simulink model "wlan_phy_rx_pmd"  (Simulink 7.8)
settings: Solver=VariableStepDiscrete  SolverMode=SingleTasking  StartTime=0.0  StopTime=rx_sim_time  RelTol=1e-3
PostLoadFcn:
  | wlan_phy_rx_init
InitFcn:
  | wlan_phy_rx_init
StartFcn:
  | wlan_phy_rx_init
values from neighbouring files (symbols this model uses):
  barker_seq20 = 0.95 * circshift(barker_seq20, [0 4]) ./ max(abs(barker_seq20))    [wlan_phy_rx_init.m]
  bit_scrambler_lfsr_bytes = sum(bit_scrambler_lfsr_bytes .* repmat(2.^[0:7], 127, 1),2)    [wlan_phy_rx_init.m]
  deinterleave_ROM = [deinterleave_ROM interleave_11n_64QAM zeros(1, 512-length(interleave_11n_64QAM))]    [wlan_phy_rx_init.m]
  longCorr_coef_i = [3*real(longCorr_coef)]    [wlan_phy_rx_init.m]
  longCorr_coef_q = [3*imag(longCorr_coef)]    [wlan_phy_rx_init.m]
  MAX_CP_LEN = 32    [wlan_phy_rx_init.m]
  MAX_NCBPS = NCBPS_11n_64QAM    (= 312)    [wlan_phy_rx_init.m]
  MAX_NUM_BYTES = 2^16-1    (= 65535)    [wlan_phy_rx_init.m]
  MAX_NUM_SC = 64    [wlan_phy_rx_init.m]
  MAX_NUM_SYMS = 600    [wlan_phy_rx_init.m]
  NCBPS_11a_BPSK = 48    [wlan_phy_rx_init.m]
  NDBPS_11a_BPSK12 = 24    [wlan_phy_rx_init.m]
  PHY_SIGNAL_MIN_LEN = 14    [wlan_phy_rx_init.m]
  preFFT_sampBuff_numSamps = 4*MAX_NUM_SC    (= 256)    [wlan_phy_rx_init.m]
  rx_sim_sig_samp_time = 8    [wlan_phy_rx_init.m]
  scr_ind_rev = zeros(1,128)    [wlan_phy_rx_init.m]
undefined symbols (not defined in the file or in its folder): gcbh, mcs_rom_11ag, mcs_rom_11n, xlBlockLoadCallback, xlGetNormalizedPeriod, xlipgetpartsetting
libraries: xbsIndex_r4 x3862, simulink x1, xbsEDKLib_r4 x1
(part 5 of 23)

== system wlan_phy_rx_pmd/DSSS Rx/SIGNAL Dec ==
  note: LENGTH*8 + 6 - 1:
      | Length in usec = Number of bits
      | *8: Convert to bytes
      | +6: Add SIGNAL, SERVICE and CRC bytes
      | -1: Downstream logic requires zero-indexed length
      | to compare vs. byte index
  Byte : Inport
  Byte Valid : Inport  Port=2
  Byte Ind : Inport  Port=3
  CRC Calc : Inport  Port=4
  AddSub : xbsIndex_r4/AddSub  mode=Addition  use_carryin=off  use_carryout=off  en=off  latency=0  precision=User Defined  arith_type=Unsigned  n_bits=ceil(log2(MAX_NUM_BYTES))  bin_pt=0  quantization=Truncate  overflow=Wrap  dbl_ovrd=off  use_behavioral_HDL=off  hw_selection=Fabric  pipelined=off  block_type=addsub
  Concat : xbsIndex_r4/Concat  num_inputs=2  dbl_ovrd=off  block_type=concat
  Concat1 : xbsIndex_r4/Concat  num_inputs=2  dbl_ovrd=off  block_type=concat
  Constant1 : xbsIndex_r4/Constant  const=2  gui_display_data_type=Fixed-point  arith_type=Unsigned  n_bits=2  bin_pt=0  preci_type=Single  exp_width=8  frac_width=24  explicit_period=off  period=1  equ=P=C  opselect=C  inp2=PCIN>>17  opr=+  inp1=P  carry=CIN  dbl_ovrd=off  block_type=constant
  Constant2 : xbsIndex_r4/Constant  const=0  gui_display_data_type=Fixed-point  arith_type=Unsigned  n_bits=2  bin_pt=0  preci_type=Single  exp_width=8  frac_width=24  explicit_period=off  period=1  equ=P=C  opselect=C  inp2=PCIN>>17  opr=+  inp1=P  carry=CIN  dbl_ovrd=off  block_type=constant
  Constant3 : xbsIndex_r4/Constant  const=10  gui_display_data_type=Fixed-point  arith_type=Unsigned  n_bits=8  bin_pt=0  preci_type=Single  exp_width=8  frac_width=24  explicit_period=off  period=1  equ=P=C  opselect=C  inp2=PCIN>>17  opr=+  inp1=P  carry=CIN  dbl_ovrd=off  block_type=constant
  Constant4 : xbsIndex_r4/Constant  const=3  gui_display_data_type=Fixed-point  arith_type=Unsigned  n_bits=2  bin_pt=0  preci_type=Single  exp_width=8  frac_width=24  explicit_period=off  period=1  equ=P=C  opselect=C  inp2=PCIN>>17  opr=+  inp1=P  carry=CIN  dbl_ovrd=off  block_type=constant
  Constant6 : xbsIndex_r4/Constant  const=4  gui_display_data_type=Fixed-point  arith_type=Unsigned  n_bits=3  bin_pt=0  preci_type=Single  exp_width=8  frac_width=24  explicit_period=off  period=1  equ=P=C  opselect=C  inp2=PCIN>>17  opr=+  inp1=P  carry=CIN  dbl_ovrd=off  block_type=constant
  Constant7 : xbsIndex_r4/Constant  const=5  gui_display_data_type=Fixed-point  arith_type=Unsigned  n_bits=3  bin_pt=0  preci_type=Single  exp_width=8  frac_width=24  explicit_period=off  period=1  equ=P=C  opselect=C  inp2=PCIN>>17  opr=+  inp1=P  carry=CIN  dbl_ovrd=off  block_type=constant
  Constant9 : xbsIndex_r4/Constant  const=5  gui_display_data_type=Fixed-point  arith_type=Unsigned  n_bits=3  bin_pt=0  preci_type=Single  exp_width=8  frac_width=24  explicit_period=off  period=8  equ=P=C  opselect=C  inp2=PCIN>>17  opr=+  inp1=P  carry=CIN  dbl_ovrd=off  block_type=constant
  DOUT{,2,4..5} (4 alike) : xbsIndex_r4/Register  init=0  rst=on  en=on  dbl_ovrd=off  block_type=register
  DOUT3 : xbsIndex_r4/Register  init=4  rst=on  en=on  dbl_ovrd=off  block_type=register
  DSSS SIGNAL Decode : Scope  NumInputPorts=7
      settings: TimeRange=14500  YMin=0~0~972.8~0.95~-1~-1~-1  YMax=10~1~1075.2~1.05~1~1~1  SaveName=ScopeData44
  Delay1 : xbsIndex_r4/Delay  rst=off  infoeditControl=Selection of Reset will increase slice count due to use of real FFs and instead of SRLs  en=off  latency=1  dbl_ovrd=off  reg_retiming=off  block_type=delay
  Display : Display
  Display1 : Display
  Gateway Out{,1..7} (8 alike) : xbsIndex_r4/Gateway Out  inherit_from_input=off  hdl_port=off  timing_constraint=None  locs_specified=off  LOCs={}  UseAsDAC=off  DACChannel='1'  block_type=gatewayout
  Inverter1 : xbsIndex_r4/Inverter  en=off  latency=0  dbl_ovrd=off  block_type=inv
  Length Check : Subsystem
  Logical{,1..3,7..10} (8 alike) : xbsIndex_r4/Logical  logical_function=AND  inputs=2  en=off  latency=0  precision=Full  arith_type=Unsigned  n_bits=16  bin_pt=0  align_bp=on  dbl_ovrd=off  block_type=logical
  Logical6 : xbsIndex_r4/Logical  logical_function=OR  inputs=3  en=off  latency=0  precision=Full  arith_type=Unsigned  n_bits=16  bin_pt=0  align_bp=on  dbl_ovrd=off  block_type=logical
  Relational{1..2,4,6..7} (5 alike) : xbsIndex_r4/Relational  mode=a=b  en=off  latency=0  dbl_ovrd=off  block_type=relational
  Relational3 : xbsIndex_r4/Relational  mode=a!=b  en=off  latency=0  dbl_ovrd=off  block_type=relational
  Relational8 : xbsIndex_r4/Relational  mode=a!=b  en=off  latency=0  dbl_ovrd=off  block_type=relational
  Shift : xbsIndex_r4/Shift  shift_dir=Right  shift_bits=3  en=off  latency=0  precision=Full  arith_type="Signed  (2's comp)"  n_bits=16  bin_pt=14  quantization=Truncate  overflow=Wrap  dbl_ovrd=off  block_type=shift
  LENGTH : Outport
  Valid Header : Outport  Port=2
  Error : Outport  Port=3
  wires:
  Constant2/1 -> Relational2/2
  Relational1/1 -> Logical/2
  Relational2/1 -> Logical2/2
  Logical/1 -> DOUT2/3
  Logical2/1 -> DOUT/3
  Byte/1 -> DOUT/1, DOUT2/1, DOUT3/1, DOUT4/1, DOUT5/1, Gateway Out2/1
  [DSSS_RX_RESET] -> DOUT/2, DOUT2/2, DOUT3/2, DOUT4/2, DOUT5/2
  Byte Valid/1 -> Logical2/1, Logical/1, Logical3/1, Logical8/1, Logical7/1, Gateway Out3/1
  Constant1/1 -> Relational1/2
  Byte Ind/1 -> Relational1/1, Relational4/1, Relational7/1, Relational6/1, Relational2/1
  DOUT/1 -> Relational3/1, Gateway Out1/1
  Constant3/1 -> Relational3/2
  Relational4/1 -> Logical3/2
  Constant4/1 -> Relational4/2
  Logical3/1 -> DOUT3/3
  DOUT2/1 -> Concat/2
  DOUT3/1 -> Concat/1
  Concat/1 -> Gateway Out/1, Shift/1, Gateway Out6/1, Length Check/1(Len)
  Length Check/1(Invalid) -> Logical6/2
  Relational3/1 -> Logical6/1
  Gateway Out/1 -> Display/1
  Gateway Out1/1 -> Display1/1
  Constant6/1 -> Relational6/2
  Logical8/1 -> DOUT4/3, Delay1/1
  Relational6/1 -> Logical7/2
  Relational7/1 -> Logical8/2
  Constant7/1 -> Relational7/2
  DOUT4/1 -> Concat1/1
  DOUT5/1 -> Concat1/2
  Concat1/1 -> Relational8/1
  CRC Calc/1 -> Relational8/2
  Relational8/1 -> Logical9/1, Gateway Out7/1
  Delay1/1 -> Logical9/2, Logical10/2, Logical1/2
  Logical9/1 -> Logical6/3
  Logical7/1 -> DOUT5/3
  Constant9/1 -> AddSub/2
  AddSub/1 -> LENGTH/1
  Logical6/1 -> Inverter1/1, Logical1/1
  Inverter1/1 -> Logical10/1
  Logical10/1 -> Valid Header/1, Gateway Out4/1
  Logical1/1 -> Error/1, Gateway Out5/1
  Shift/1 -> AddSub/1
  Gateway Out2/1 -> DSSS SIGNAL Decode/1
  Gateway Out3/1 -> DSSS SIGNAL Decode/2
  Gateway Out4/1 -> DSSS SIGNAL Decode/6
  Gateway Out5/1 -> DSSS SIGNAL Decode/7
  Gateway Out6/1 -> DSSS SIGNAL Decode/3
  Gateway Out7/1 -> DSSS SIGNAL Decode/4

== system wlan_phy_rx_pmd/DSSS Rx/Sym Sync ==
  En : Inport
  Rx I : Inport  Port=2
  Rx Q : Inport  Port=3
  ASR : xbsIndex_r4/Addressable Shift Register  infer_latency=on  depth=2  initVector=[0]  en=off  dbl_ovrd=off  use_behavioral_HDL=off  Optimization=Resource  block_type=addrsr
      settings: infoedit="Delay of configurable length.  Any element in the delay line can be addressed and driven onto the output port.<br><br>Hardware notes: This block is implemented using SRL16s. If Virtex-4 or Spartan-3 device is used, multiple SRLC16s are cascaded together."
  Abs : Subsystem
  DSSS Corr : Scope  NumInputPorts=7
      settings: TimeRange=14500  YMin=-1~-7.5~0~0~0~0~-1  YMax=1~7.5~35~1~1~1~1
  Display : Display
  Display1 : Display
  Down Sample1 : xbsIndex_r4/Down Sample  sample_ratio=8  sample_phase="Last Value of Frame  (most efficient)"  en=off  latency=1  dbl_ovrd=off  block_type=dsamp
  Down Sample2 : xbsIndex_r4/Down Sample  sample_ratio=8  sample_phase="Last Value of Frame  (most efficient)"  en=off  latency=1  dbl_ovrd=off  block_type=dsamp
  "FIR Compiler 6.3 " : xbsIndex_r4/FIR Compiler 6.3   coefficientvector=fliplr(barker_seq20)  coefficient_sets=1  coefficient_reload=off  filter_type=Single_Rate  rate_change_type=Integer  interpolation_rate=1  decimation_rate=1  zero_pack_factor=1  channel_sequence=Basic  number_channels=1  pattern_list='P4-0,P4-1,P4-2,P4-3,P4-4'  number_paths=2  ratespecification=Maximum_Possible  sampleperiod=8  hardwareoversamplingrate=1  coefficient_sign=Signed  quantization=Maximize_Dynamic_Range  coefficient_width=16  bestprecision=on  coefficient_fractional_bits=15  coefficient_structure=Inferred  output_rounding_mode=Truncate_LSBs  output_width=16  filter_architecture=Systolic_Multiply_Accumulate  optimization_goal=Area  data_buffer_type=Automatic  coefficient_buffer_type=Automatic  input_buffer_type=Automatic  output_buffer_type=Automatic  preference_for_other_storage=Automatic  multi_column_support=Automatic  columnconfig='20'  inter_column_pipe_length=4  data_has_tlast=Not_Required  m_data_has_tready=off  s_data_has_fifo=off  s_data_has_tuser=Not_Required  m_data_has_tuser=Not_Required  s_config_sync_mode=On_Vector  s_config_method=Single  num_reload_slots=1  has_aclken=off  has_aresetn=off  infoeditControl=ARESETn must be asserted for a minimum of 2 cycles  trim_axipin_name=on  passband_min=0.0  passband_max=0.5  stopband_min=0.5  stopband_max=1.0  filter_selection=1  gui_behaviour=Sysgen_GUI  data_sign=Signed  data_width=16  data_fractional_bits=0  data_tuser_width=1  wrapper_available=true  ip_wrap_arbitrary_binary_point=true  ip_name=FIR Compiler  ip_version=6.3  dsptool_ready=true  ipcore_usecache=true  ipcore_useipmodelcache=true  ipcore_xco_need_fpga_part=true  port_translation_map={ 'aclken' => 'en', 'aresetn' => 'rst' }  run_core_at_system_period=true  ipcore_fpga_part=xlipgetpartsetting(gcb, {'virtex6', 'xc6vlx75t', '-3', 'ff784'})  block_type=fir_compiler_v6_3
      settings: infoedit=Maximum Possible : Maximizes oversampling rate. Abstracted input handshake (no s_data_tvalid) and automatic rate propagation.<br>Hardware Oversampling Rate : Specifies oversampling rate relative to the input sample period. Abstracted input handshake (no s_data_tvalid) and automatic rate propagation.<br>Sample Period : Specifies absolute oversampling rate. No input handshake abstraction (exposes s_data_tvalid) and no rate propagation.
  Gateway Out{,1..7} (8 alike) : xbsIndex_r4/Gateway Out  inherit_from_input=off  hdl_port=off  timing_constraint=None  locs_specified=off  LOCs={}  UseAsDAC=off  DACChannel='1'  block_type=gatewayout
  Posedge : Subsystem
  Relational : xbsIndex_r4/Relational  mode=a<b  en=off  latency=0  dbl_ovrd=off  block_type=relational
  S-R Latch : Subsystem
  Sym Match : Subsystem
  Sym Sync Timeout : Subsystem
  Sym : Outport
  Timeout : Outport  Port=2
  wires:
  Rx Q/1 -> Gateway Out/1, "FIR Compiler 6.3 "/2
  Gateway Out/1 -> DSSS Corr/1
  "FIR Compiler 6.3 "/2 -> Abs/1(Valid)
  "FIR Compiler 6.3 "/3 -> Abs/2(I)
  "FIR Compiler 6.3 "/4 -> Abs/3(Q), Gateway Out1/1
  Gateway Out1/1 -> DSSS Corr/2
  Abs/1(A) -> Relational/2, Gateway Out2/1
  [regRx_DSSS_RX_CODE_CORR_THRESH] -> Down Sample2/1
  Rx I/1 -> "FIR Compiler 6.3 "/1
  Relational/1 -> ASR/1
  Gateway Out2/1 -> DSSS Corr/3
  Gateway Out3/1 -> Display/1
  [regRx_DSSS_RX_DESPREAD_DLY] -> Down Sample1/1
  Gateway Out5/1 -> DSSS Corr/4
  ? -> DSSS Corr/5
  Gateway Out6/1 -> DSSS Corr/6
  Gateway Out7/1 -> DSSS Corr/7
  Down Sample1/1 -> ASR/2, Gateway Out4/1
  Down Sample2/1 -> Relational/1, Gateway Out3/1
  ASR/1 -> Sym Match/1(Corr)
  En/1 -> Sym Match/2(En), Gateway Out6/1, Sym Sync Timeout/2(Det)
  Sym Match/1(Sym) -> Gateway Out7/1, Posedge/1(D)
  Gateway Out4/1 -> Display1/1
  Posedge/1(Q) -> S-R Latch/1(S)
  [DSSS_RX_RESET] -> S-R Latch/2(R)
  S-R Latch/1(Q) -> Sym/1, Sym Sync Timeout/1(Sym)
  Sym Sync Timeout/1(Timeout) -> Timeout/1, Gateway Out5/1

== system wlan_phy_rx_pmd/Detect & Decode/De-Interleave & De-Puncture ==
  note: This RAM contains one OFDM symbol's worth of coded bits, represented as log-likelihood ratios (LLR).
      | These soft bits are written to the RAM in pairs (except for BPSK) in linear order. Once a full symbol's
      | worth of bits is written the same bits are then read in de-interleaved order. The bits are read in pairs and
      | fed directly into the rate 1/2 Viterbi decoder. Operating on pairs-of-bits here significantly decreases the latency
      | of the de-interleaving process and is necessary to finish decoding 64QAM 3/4 payloads inside the
      | alloted 6usec post-Rx time.
      | De-puncturing is realized by setting the appropriate LLR output (A or B) to 0, indicating a zero-confidence
      | value for the decoder. The Read Address Generator logic tracks which bit positions should have these
      | inserted zero-confidence values.
  LLR A : Inport
  LLR B : Inport  Port=2
  tvalid : Inport  Port=3
  mod_sel : Inport  Port=4
  Demod cfg : Inport  Port=5
  Reset : Inport  Port=6
  DeInt : Scope  NumInputPorts=9
      settings: TimeRange=70.99443917115605  YMin=-1~0.95~-1~-1~-1~45.6~-1~-4.2~-1  YMax=1~1.05~1~1~1~50.4~1~-3.8~1  SaveName=ScopeData7
  Delay3 : xbsIndex_r4/Delay  rst=off  infoeditControl=Selection of Reset will increase slice count due to use of real FFs and instead of SRLs  en=off  latency=1  dbl_ovrd=off  reg_retiming=off  block_type=delay
  Gateway Out{1..9} (9 alike) : xbsIndex_r4/Gateway Out  inherit_from_input=off  hdl_port=off  timing_constraint=None  locs_specified=off  LOCs={}  UseAsDAC=off  DACChannel='1'  block_type=gatewayout
  Inverter1 : xbsIndex_r4/Inverter  en=off  latency=0  dbl_ovrd=off  block_type=inv
      settings: xl_area=[0 0 0 0 0 0 0]  block_version=VER_STRING_GOES_HERE
  LLR-per-bit RAM : xbsIndex_r4/Dual Port RAM  depth=2^(ceil(log2(MAX_NCBPS)))  initVector=0  distributed_mem=Block RAM  init_a=0  init_b=0  rst_a=on  rst_b=on  en_a=off  en_b=off  latency=1  write_mode_A=Read After Write  write_mode_B=Read After Write  dbl_ovrd=off  optimize=Area  block_type=dpram
  Logical3 : xbsIndex_r4/Logical  logical_function=OR  inputs=2  en=off  latency=0  precision=Full  arith_type=Unsigned  n_bits=16  bin_pt=0  align_bp=on  dbl_ovrd=off  block_type=logical
  Logical4 : xbsIndex_r4/Logical  logical_function=OR  inputs=2  en=off  latency=0  precision=Full  arith_type=Unsigned  n_bits=16  bin_pt=0  align_bp=on  dbl_ovrd=off  block_type=logical
  Mux1 : xbsIndex_r4/Mux  inputs=2  en=off  latency=0  precision=Full  arith_type=Unsigned  n_bits=16  bin_pt=14  quantization=Truncate  overflow=Wrap  dbl_ovrd=off  block_type=mux
  Mux2 : xbsIndex_r4/Mux  inputs=2  en=off  latency=0  precision=Full  arith_type=Unsigned  n_bits=16  bin_pt=14  quantization=Truncate  overflow=Wrap  dbl_ovrd=off  block_type=mux
  Read Addr Gen : Subsystem
  Write Addr Gen : Subsystem
  b0 : xbsIndex_r4/Slice  nbits=1  boolean_output=on  mode=Lower Bit Location + Width  bit1=0  base1=MSB of Input  bit0=0  base0=LSB of Input  dbl_ovrd=off  block_type=slice
  " LLR A" : Outport
  " LLR B" : Outport  Port=2
  " tvalid" : Outport  Port=3
  tag scope: CS_DeInt_ModSel=global, CS_DeInt_RdAddr=global, CS_DeInt_Reset=global, CS_DeInt_Stuff0=global, CS_DeInt_Vin=global, CS_DeInt_Vout=global, CS_DeInt_WrAddr=global, CS_DeInt_WrEn=global, CS_Deint_Addr_A=global, CS_Deint_Wen=global
  wires:
  tvalid/1 -> Write Addr Gen/1(tvalid), Gateway Out5/1  "tvalid in"
  mod_sel/1 -> Read Addr Gen/2(mod_sel), Gateway Out1/1  "mod_sel"
  Reset/1 -> Write Addr Gen/2(Reset), Read Addr Gen/3(Reset), Gateway Out9/1
  Write Addr Gen/4(Block Wr Done) -> Read Addr Gen/1(Block Wr Done), Gateway Out4/1
  Gateway Out5/1 -> DeInt/1  "tvalid in"
  Gateway Out1/1 -> DeInt/2  "mod_sel"
  Gateway Out2/1 -> DeInt/5  "Addr A"
  Gateway Out3/1 -> DeInt/3  "Wr En"
  Gateway Out4/1 -> DeInt/4  "Block Wr Done"
  Gateway Out6/1 -> DeInt/6  "Addr B"
  Gateway Out7/1 -> DeInt/7  "tvalid out"
  Gateway Out8/1 -> DeInt/8  "Mem out"
  Gateway Out9/1 -> DeInt/9  "Reset In"
  LLR-per-bit RAM/2 -> " LLR B"/1
  Mux2/1 -> Gateway Out2/1, LLR-per-bit RAM/1, [CS_Deint_Addr_A]
  Mux1/1 -> LLR-per-bit RAM/4, Gateway Out6/1
  Write Addr Gen/3(Wr En) -> Mux1/1, Mux2/1, LLR-per-bit RAM/6, LLR-per-bit RAM/3, [CS_Deint_Wen], Gateway Out3/1
  Write Addr Gen/1(Wr Addr A) -> Mux2/3
  Write Addr Gen/2(Wr Addr B) -> Mux1/3
  LLR A/1 -> LLR-per-bit RAM/2
  LLR B/1 -> LLR-per-bit RAM/5
  LLR-per-bit RAM/1 -> Gateway Out8/1, " LLR A"/1
  Read Addr Gen/1(Rd Addr A) -> Mux2/2
  Read Addr Gen/2(Rd Addr B) -> Mux1/2
  Read Addr Gen/5(Vout) -> Delay3/1, Inverter1/1
  Delay3/1 -> " tvalid"/1, Gateway Out7/1
  Read Addr Gen/3(Skip A) -> Logical3/1
  Read Addr Gen/4(Skip B) -> Logical4/1
  Logical3/1 -> LLR-per-bit RAM/7
  Logical4/1 -> LLR-per-bit RAM/8
  Inverter1/1 -> Logical4/2, Logical3/2
  Demod cfg/1 -> b0/1
  b0/1 -> Read Addr Gen/4(SIGNAL sym), Write Addr Gen/3(SIGNAL Sym)  "SIGNAL sym"
  [OFDM_RX_DATA_Code_Rate] -> Read Addr Gen/5(rate_sel)

== system wlan_phy_rx_pmd/Detect & Decode/Decode ==
  note: Required to avoid combinational loop
      | back to BB reset input
  LLR A : Inport
  LLR B : Inport  Port=2
  Vin : Inport  Port=3
  Start : Inport  Port=4
  Reset : Inport  Port=5
  Assert11 : xbsIndex_r4/Assert  assert_type=off  type_source=Explicitly  gui_display_data_type=Fixed-point  arith_type="Signed  (2's comp)"  n_bits=16  bin_pt=14  preci_type=Single  exp_width=8  frac_width=24  assert_rate=on  rate_source=Explicitly  period=1  output_port=on  block_type=assert
      settings: block_version=VER_STRING_GOES_HERE
  Byte Count : Subsystem
  Control : Subsystem
  Convert3 : xbsIndex_r4/Convert  gui_display_data_type=Boolean  arith_type=Boolean  n_bits=1  bin_pt=0  float_type=Single  exp_bits=8  fraction_bits=24  quantization=Truncate  overflow=Wrap  en=off  latency=0  dbl_ovrd=off  pipeline=off  block_type=convert
  Decoder I/O : Scope  NumInputPorts=12
      settings: TimeRange=9204  YMin=-7.5~-7.5~0~0~0~0~0~-1~-1~0~-5~-5  YMax=7.5~7.5~1~1~1~1~1~1~1~7.5~5~5  SaveName=ScopeData3
  Delay : xbsIndex_r4/Delay  rst=off  infoeditControl=Selection of Reset will increase slice count due to use of real FFs and instead of SRLs  en=off  latency=1  dbl_ovrd=off  reg_retiming=off  block_type=delay
  Endian Swap : Subsystem
  Gateway Out{1..12} (12 alike) : xbsIndex_r4/Gateway Out  inherit_from_input=off  hdl_port=off  timing_constraint=None  locs_specified=off  LOCs={}  UseAsDAC=off  DACChannel='1'  block_type=gatewayout
  Logical1 : xbsIndex_r4/Logical  logical_function=OR  inputs=3  en=off  latency=0  precision=Full  arith_type=Unsigned  n_bits=16  bin_pt=0  align_bp=on  dbl_ovrd=off  block_type=logical
  Logical2 : xbsIndex_r4/Logical  logical_function=OR  inputs=2  en=off  latency=0  precision=Full  arith_type=Unsigned  n_bits=16  bin_pt=0  align_bp=on  dbl_ovrd=off  block_type=logical
  Register : xbsIndex_r4/Register  init=0  rst=off  en=off  dbl_ovrd=off  block_type=register
  Register4 : xbsIndex_r4/Register  init=0  rst=off  en=off  dbl_ovrd=off  block_type=register
  Register5 : xbsIndex_r4/Register  init=0  rst=off  en=off  dbl_ovrd=off  block_type=register
  Reinterpret : xbsIndex_r4/Reinterpret  force_arith_type=on  arith_type=Unsigned  force_bin_pt=on  bin_pt=0  block_type=reinterpret
  Reinterpret2 : xbsIndex_r4/Reinterpret  force_arith_type=on  arith_type=Unsigned  force_bin_pt=on  bin_pt=0  block_type=reinterpret
  SIGNAL & HT-SIG Decode : Subsystem
  Safe ToUint8 : Subsystem
  Stretch : Subsystem
  Viberbi Decoder : xbsIndex_r4/Black Box  init_code=vb_decoder_top_config  sim_method=ISE Simulator  verbose=off  block_type=blackbox2
  Byte : Outport
  Byte Valid : Outport  Port=2
  Byte Index : Outport  Port=3
  Last Byte : Outport  Port=4
  " Reset" : Outport  Port=5
  tag scope: CS_DEC_BYTE_INDEX=global, CS_DEC_BYTE_OUT=global, CS_DEC_BYTE_VALID_OUT=global, CS_DEC_END=global, CS_DEC_LLR_b0=global, CS_DEC_LLR_b1=global, CS_DEC_RESET=global, CS_DEC_START=global, CS_DEC_VIN=global, CS_DEC_VOUT=global
  wires:
  Register/1 -> Reinterpret/1, Gateway Out11/1, [CS_DEC_LLR_b1]
  Register4/1 -> Reinterpret2/1, Gateway Out10/1, [CS_DEC_LLR_b0]
  Register5/1 -> Control/1(dec_vin)
  Viberbi Decoder/2 -> Convert3/1, Control/5(dec_vout)
  Viberbi Decoder/3 -> Safe ToUint8/1(A)
  Start/1 -> Logical2/1, Byte Count/2(Decode Start), Control/3(Start)
  Safe ToUint8/1(B) -> Endian Swap/1(B)
  Byte Count/2(Byte Index) -> Byte Index/1, [CS_DEC_BYTE_INDEX], Gateway Out5/1, SIGNAL & HT-SIG Decode/1(byte_ind)
  Gateway Out1/1 -> Decoder I/O/11  "Vout"
  Gateway Out5/1 -> Decoder I/O/10  "Byte Index"
  Gateway Out6/1 -> Decoder I/O/9  "Last Byte"
  Gateway Out4/1 -> Decoder I/O/6  "LLR Valid"
  Gateway Out2/1 -> Decoder I/O/7  "Start"
  Gateway Out3/1 -> Decoder I/O/8  "End"
  Assert11/1 -> Logical2/2, Stretch/1(D)
  Logical1/1 -> Assert11/1
  Gateway Out8/1 -> Decoder I/O/12  "Reset Out"
  Logical2/1 -> SIGNAL & HT-SIG Decode/4(rst)
  Vin/1 -> Register5/1
  LLR B/1 -> Register4/1
  LLR A/1 -> Register/1
  Byte Count/3(Last Byte) -> Last Byte/1, Delay/1, Gateway Out6/1
  SIGNAL & HT-SIG Decode/1(Last Byte Ind) -> Byte Count/3(Last Byte Ind), Control/4(Num Bytes)
  Byte Count/1(" Byte Valid") -> Byte Valid/1, [CS_DEC_BYTE_VALID_OUT], Gateway Out1/1, SIGNAL & HT-SIG Decode/2(byte_valid)
  Stretch/1(Q) -> " Reset"/1, Gateway Out8/1
  Convert3/1 -> Byte Count/1(Byte Valid), [CS_DEC_VOUT]
  Gateway Out9/1 -> Decoder I/O/5  "Reset#"
  Delay/1 -> Logical1/1
  Gateway Out10/1 -> Decoder I/O/1  "LLR B0"
  Gateway Out11/1 -> Decoder I/O/2  "LLR B1"
  Gateway Out12/1 -> Decoder I/O/3  "Early Trace 1"
  Control/1(vin) -> Viberbi Decoder/3, Gateway Out4/1, [CS_DEC_VIN]
  Control/2(rst#) -> Viberbi Decoder/4, Gateway Out9/1, [CS_DEC_RESET]
  Control/3(start) -> Viberbi Decoder/5, Gateway Out2/1, [CS_DEC_START]
  Control/4(pkt_end) -> Viberbi Decoder/6, Gateway Out3/1, [CS_DEC_END]
  Control/5(early_trace_1) -> Viberbi Decoder/7, Gateway Out12/1
  Reset/1 -> Control/2(Reset)
  Reinterpret/1 -> Viberbi Decoder/1
  Reinterpret2/1 -> Viberbi Decoder/2
  Control/6(early_trace_2) -> Viberbi Decoder/8, Gateway Out7/1
  Gateway Out7/1 -> Decoder I/O/4  "Early Trace 2"
  Endian Swap/1(I) -> Byte/1, SIGNAL & HT-SIG Decode/3(byte), [CS_DEC_BYTE_OUT]
  [RX_GLOBAL_RESET] -> Logical1/2
  SIGNAL & HT-SIG Decode/2(Reset PHY) -> Logical1/3

== system wlan_phy_rx_pmd/Detect & Decode/Descramble ==
  note: SERVICE field starts after
      | SIGNAL fields, byte 3 for 11a/g
      | byte 9 for 11n/ac
  Byte : Inport
  Byte Valid : Inport  Port=2
  Byte Index : Inport  Port=3
  Last Byte : Inport  Port=4
  Constant : xbsIndex_r4/Constant  const=3  gui_display_data_type=Fixed-point  arith_type=Unsigned  n_bits=4  bin_pt=0  preci_type=Single  exp_width=8  frac_width=24  explicit_period=off  period=1  equ=P=C  opselect=C  inp2=PCIN>>17  opr=+  inp1=P  carry=CIN  dbl_ovrd=off  block_type=constant
  Constant2 : xbsIndex_r4/Constant  const=9  gui_display_data_type=Fixed-point  arith_type=Unsigned  n_bits=4  bin_pt=0  preci_type=Single  exp_width=8  frac_width=24  explicit_period=off  period=1  equ=P=C  opselect=C  inp2=PCIN>>17  opr=+  inp1=P  carry=CIN  dbl_ovrd=off  block_type=constant
  DOUT : xbsIndex_r4/Register  init=0  rst=off  en=on  dbl_ovrd=off  block_type=register
  Delay{,1,3..4} (4 alike) : xbsIndex_r4/Delay  rst=off  infoeditControl=Selection of Reset will increase slice count due to use of real FFs and instead of SRLs  en=off  latency=3  dbl_ovrd=off  reg_retiming=off  block_type=delay
  Descram : Scope  NumInputPorts=6
      settings: TimeRange=10000  YMin=0~0~32.5~-1~-1~0  YMax=1~275~52.5~1~1~250  SaveName=ScopeData18
  Descram Byte Calc : Subsystem
  Gateway Out{1..9} (9 alike) : xbsIndex_r4/Gateway Out  inherit_from_input=off  hdl_port=off  timing_constraint=None  locs_specified=off  LOCs={}  UseAsDAC=off  DACChannel='1'  block_type=gatewayout
  Logical1 : xbsIndex_r4/Logical  logical_function=XOR  inputs=2  en=off  latency=0  precision=Full  arith_type=Unsigned  n_bits=16  bin_pt=0  align_bp=on  dbl_ovrd=off  block_type=logical
  Mux : xbsIndex_r4/Mux  inputs=2  en=off  latency=0  precision=Full  arith_type=Unsigned  n_bits=16  bin_pt=14  quantization=Truncate  overflow=Wrap  dbl_ovrd=off  block_type=mux
  To Workspace : ToWorkspace  VariableName=byteout  SampleTime=-1  SaveFormat=Array
  To Workspace1 : ToWorkspace  VariableName=byteout_valid  SampleTime=-1  SaveFormat=Array
  To Workspace2 : ToWorkspace  VariableName=byteout_index  SampleTime=-1  SaveFormat=Array
  " Byte" : Outport
  " Byte Valid" : Outport  Port=2
  " Byte Index" : Outport  Port=3
  " Last Byte" : Outport  Port=4
  wires:
  Gateway Out4/1 -> Descram/6  "Registered Output Byte"
  DOUT/1 -> Gateway Out4/1
  Delay/1 -> Logical1/1, Gateway Out9/1
  Gateway Out1/1 -> Descram/3  "Byte Index"
  Gateway Out6/1 -> Descram/2  "Byte Out"
  Gateway Out5/1 -> Descram/1  "Byte Valid"
  Delay4/1 -> Gateway Out1/1, " Byte Index"/1, Gateway Out7/1
  Delay3/1 -> Gateway Out5/1, " Byte Valid"/1, DOUT/2, Gateway Out3/1
  Logical1/1 -> " Byte"/1, Gateway Out6/1, DOUT/1, Gateway Out2/1
  Descram Byte Calc/1(Descram Val) -> Logical1/2, Gateway Out8/1
  Byte/1 -> Descram Byte Calc/1(data_byte), Delay/1
  Byte Index/1 -> Descram Byte Calc/3(data_ind), Delay4/1
  Byte Valid/1 -> Descram Byte Calc/2(data_valid), Delay3/1
  Last Byte/1 -> Delay1/1
  Delay1/1 -> " Last Byte"/1
  Gateway Out3/1 -> To Workspace1/1
  Gateway Out2/1 -> To Workspace/1
  Gateway Out7/1 -> To Workspace2/1
  Gateway Out8/1 -> Descram/4  "Descram Byte"
  Gateway Out9/1 -> Descram/5  "Input Byte"
  [RX_PHY_MODE_11N_AC] -> Mux/1
  Mux/1 -> Descram Byte Calc/4(Svc byte ind)
  Constant/1 -> Mux/2
  Constant2/1 -> Mux/3

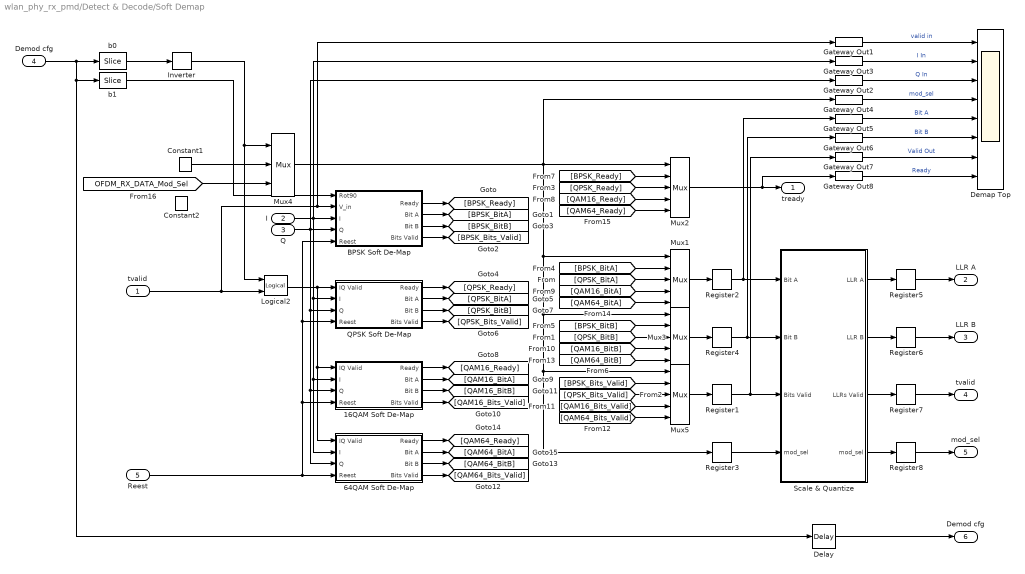
== system wlan_phy_rx_pmd/Detect & Decode/Soft Demap ==
  "tvalid " : Inport
  I : Inport  Port=2
  Q : Inport  Port=3
  Demod cfg : Inport  Port=4
  Reest : Inport  Port=5
  16QAM Soft De-Map : Subsystem
  64QAM Soft De-Map : Subsystem
  BPSK Soft De-Map : Subsystem
  Constant1 : xbsIndex_r4/Constant  const=0  gui_display_data_type=Fixed-point  arith_type=Unsigned  n_bits=2  bin_pt=0  preci_type=Single  exp_width=8  frac_width=24  explicit_period=off  period=1  equ=P=C  opselect=C  inp2=PCIN>>17  opr=+  inp1=P  carry=CIN  dbl_ovrd=off  block_type=constant
      settings: block_version=VER_STRING_GOES_HERE
  Constant2 : xbsIndex_r4/Constant  const=1  gui_display_data_type=Fixed-point  arith_type=Unsigned  n_bits=2  bin_pt=0  preci_type=Single  exp_width=8  frac_width=24  explicit_period=off  period=1  equ=P=C  opselect=C  inp2=PCIN>>17  opr=+  inp1=P  carry=CIN  dbl_ovrd=off  block_type=constant
      settings: block_version=VER_STRING_GOES_HERE
  Delay : xbsIndex_r4/Delay  rst=off  infoeditControl=Selection of Reset will increase slice count due to use of real FFs and instead of SRLs  en=off  latency=3  dbl_ovrd=off  reg_retiming=off  block_type=delay
  Demap Top : Scope  NumInputPorts=8
      settings: TimeRange=20000  YMin=-0.1~-1~0~0~-1~0~-0.1~-1  YMax=1.1~1~1~1~1~1~1.1~1  SaveName=ScopeData5
  Gateway Out{1..8} (8 alike) : xbsIndex_r4/Gateway Out  inherit_from_input=off  hdl_port=off  timing_constraint=None  locs_specified=off  LOCs={}  UseAsDAC=off  DACChannel='1'  block_type=gatewayout
  Inverter : xbsIndex_r4/Inverter  en=off  latency=0  dbl_ovrd=off  block_type=inv
  Logical2 : xbsIndex_r4/Logical  logical_function=AND  inputs=2  en=off  latency=0  precision=Full  arith_type=Unsigned  n_bits=16  bin_pt=0  align_bp=on  dbl_ovrd=off  block_type=logical
  Mux{1..3,5} (4 alike) : xbsIndex_r4/Mux  inputs=4  en=off  latency=0  precision=Full  arith_type=Unsigned  n_bits=16  bin_pt=14  quantization=Truncate  overflow=Wrap  dbl_ovrd=off  block_type=mux
  Mux4 : xbsIndex_r4/Mux  inputs=2  en=off  latency=0  precision=Full  arith_type=Unsigned  n_bits=16  bin_pt=14  quantization=Truncate  overflow=Wrap  dbl_ovrd=off  block_type=mux
  QPSK Soft De-Map : Subsystem
  Register{1..8} (8 alike) : xbsIndex_r4/Register  init=0  rst=off  en=off  dbl_ovrd=off  block_type=register
  Scale & Quantize : Subsystem
  b0 : xbsIndex_r4/Slice  nbits=1  boolean_output=on  mode=Lower Bit Location + Width  bit1=0  base1=MSB of Input  bit0=0  base0=LSB of Input  dbl_ovrd=off  block_type=slice
  b1 : xbsIndex_r4/Slice  nbits=1  boolean_output=on  mode=Lower Bit Location + Width  bit1=0  base1=MSB of Input  bit0=1  base0=LSB of Input  dbl_ovrd=off  block_type=slice
  tready : Outport
  LLR A : Outport  Port=2
  LLR B : Outport  Port=3
  tvalid : Outport  Port=4
  " mod_sel" : Outport  Port=5
  " Demod cfg" : Outport  Port=6
  wires:
  "tvalid "/1 -> Logical2/2, BPSK Soft De-Map/2(V_in), Gateway Out1/1
  Mux4/1 -> Mux1/1, Mux3/1, Mux5/1, Register3/1, Gateway Out4/1, Mux2/1
  Mux2/1 -> tready/1, Gateway Out8/1
  Gateway Out2/1 -> Demap Top/3  "Q In"
  Gateway Out1/1 -> Demap Top/1  "valid in"
  Gateway Out3/1 -> Demap Top/2  "I In"
  Gateway Out4/1 -> Demap Top/4  "mod_sel"
  [OFDM_RX_DATA_Mod_Sel] -> Mux4/3
  b0/1 -> Inverter/1
  Gateway Out5/1 -> Demap Top/5  "Bit A"
  Gateway Out6/1 -> Demap Top/6  "Bit B"
  Scale & Quantize/1(LLR A) -> Register5/1
  Scale & Quantize/3(LLRs Valid) -> Register7/1
  Scale & Quantize/4(" mod_sel") -> Register8/1
  Gateway Out7/1 -> Demap Top/7  "Valid Out"
  Constant1/1 -> Mux4/2
  Register2/1 -> Gateway Out5/1, Scale & Quantize/1(Bit A)
  Register1/1 -> Scale & Quantize/3(Bits Valid), Gateway Out7/1
  Register3/1 -> Scale & Quantize/4(mod_sel)
  BPSK Soft De-Map/1(Ready) -> [BPSK_Ready]
  BPSK Soft De-Map/2(Bit A) -> [BPSK_BitA]
  BPSK Soft De-Map/3(Bit B) -> [BPSK_BitB]
  BPSK Soft De-Map/4(Bits Valid) -> [BPSK_Bits_Valid]
  QPSK Soft De-Map/1(Ready) -> [QPSK_Ready]
  QPSK Soft De-Map/2(Bit A) -> [QPSK_BitA]
  QPSK Soft De-Map/3(Bit B) -> [QPSK_BitB]
  QPSK Soft De-Map/4(Bits Valid) -> [QPSK_Bits_Valid]
  I/1 -> BPSK Soft De-Map/3(I), Gateway Out3/1, QPSK Soft De-Map/2(I), 16QAM Soft De-Map/2(I), 64QAM Soft De-Map/2(I)
  Q/1 -> BPSK Soft De-Map/4(Q), Gateway Out2/1, QPSK Soft De-Map/3(Q), 16QAM Soft De-Map/3(Q), 64QAM Soft De-Map/3(Q)
  Logical2/1 -> QPSK Soft De-Map/1(IQ Valid), 16QAM Soft De-Map/1(IQ Valid), 64QAM Soft De-Map/1(IQ Valid)
  [BPSK_Ready] -> Mux2/2
  [QPSK_Ready] -> Mux2/3
  Mux1/1 -> Register2/1
  [QPSK_BitA] -> Mux1/3
  [BPSK_BitA] -> Mux1/2
  Register4/1 -> Scale & Quantize/2(Bit B), Gateway Out6/1
  Mux3/1 -> Register4/1
  [BPSK_BitB] -> Mux3/2
  [QPSK_BitB] -> Mux3/3
  Mux5/1 -> Register1/1
  [BPSK_Bits_Valid] -> Mux5/2
  [QPSK_Bits_Valid] -> Mux5/3
  Scale & Quantize/2(LLR B) -> Register6/1
  Gateway Out8/1 -> Demap Top/8  "Ready"
  16QAM Soft De-Map/1(Ready) -> [QAM16_Ready]
  16QAM Soft De-Map/2(Bit A) -> [QAM16_BitA]
  16QAM Soft De-Map/3(Bit B) -> [QAM16_BitB]
  16QAM Soft De-Map/4(Bits Valid) -> [QAM16_Bits_Valid]
  [QAM16_Ready] -> Mux2/4
  [QAM16_BitA] -> Mux1/4
  [QAM16_BitB] -> Mux3/4
  [QAM16_Bits_Valid] -> Mux5/4
  64QAM Soft De-Map/1(Ready) -> [QAM64_Ready]
  64QAM Soft De-Map/2(Bit A) -> [QAM64_BitA]
  64QAM Soft De-Map/3(Bit B) -> [QAM64_BitB]
  64QAM Soft De-Map/4(Bits Valid) -> [QAM64_Bits_Valid]
  [QAM64_Bits_Valid] -> Mux5/5
  [QAM64_BitB] -> Mux3/5
  [QAM64_BitA] -> Mux1/5
  [QAM64_Ready] -> Mux2/5
  Register5/1 -> LLR A/1
  Register6/1 -> LLR B/1
  Register7/1 -> tvalid/1
  Register8/1 -> " mod_sel"/1
  Reest/1 -> 64QAM Soft De-Map/4(Reest), 16QAM Soft De-Map/4(Reest), QPSK Soft De-Map/4(Reest), BPSK Soft De-Map/5(Reest)
  b1/1 -> BPSK Soft De-Map/1(Rot90)
  Inverter/1 -> Mux4/1, Logical2/1
  Demod cfg/1 -> b0/1, b1/1, Delay/1
  Delay/1 -> " Demod cfg"/1

== system wlan_phy_rx_pmd/Detect & Decode/Subcarrier Map & Demod Control ==
  note: Syms 5 and 6 have no data in 11n/ac
      | (HT STF, HTLTF)
  note: OFDM Symbols in 11a/g:
      | 0: LTF (BPSK)
      | 1: LTF (BPSK)
      | 2: SIG (BPSK) 48sc
      | 3-N: Data  48sc
  note: OFDM Symbols in 11n SISO:
      | 0: L-LTF (BPSK)
      | 1: L-LTF (BPSK)
      | 2: L-SIG (BPSK) 48sc
      | 3: HT-SIG1 (QBPSK) 48sc
      | 4: HT-SIG2 (QBPSK) 48sc
      | 5: HT-STF
      | 6: HT-LFT1
      | 7-N: Data 52sc
  note: OFDM Symbols in 11ac SISO:
      | 0: L-LTF (BPSK)
      | 1: L-LTF (BPSK)
      | 2: L-SIG (BPSK) 48sc
      | 3: VHT-SIGA1 (BPSK) 48sc
      | 4: VHT-SIGA2 (QBPSK) 48sc
      | 5: VHT-STF
      | 6: VHT-LFT1
      | 7: VHT-SIGB 52sc
      | 8-N: Data 52sc
  note: Lookup tables below are 0 latency - add delays to I/Q here
      | if that changes in a future rev
  note: Capture current PHY mode decision
      | at start of each OFDM sym (subcarrier 0)
      | Every PHY mode sets subcarrier 0 to 0,
      | so one ambiguity about PHY mode
      | at subcarrier 0 is no problem
  Eq I : Inport
  Eq Q : Inport  Port=2
  iq_valid : Inport  Port=3
  sc_ind : Inport  Port=4
  Sym ind : Inport  Port=5
  Reset : Inport  Port=6
  Concat : xbsIndex_r4/Concat  num_inputs=2  dbl_ovrd=off  block_type=concat
  Constant1 : xbsIndex_r4/Constant  const=0  gui_display_data_type=Fixed-point  arith_type=Unsigned  n_bits=1  bin_pt=0  preci_type=Single  exp_width=8  frac_width=24  explicit_period=off  period=1  equ=P=C  opselect=C  inp2=PCIN>>17  opr=+  inp1=P  carry=CIN  dbl_ovrd=off  block_type=constant
  Constant2 : xbsIndex_r4/Constant  const=1  gui_display_data_type=Fixed-point  arith_type=Unsigned  n_bits=2  bin_pt=0  preci_type=Single  exp_width=8  frac_width=24  explicit_period=off  period=1  equ=P=C  opselect=C  inp2=PCIN>>17  opr=+  inp1=P  carry=CIN  dbl_ovrd=off  block_type=constant
  Constant3 : xbsIndex_r4/Constant  const=5  gui_display_data_type=Fixed-point  arith_type=Unsigned  n_bits=3  bin_pt=0  preci_type=Single  exp_width=8  frac_width=24  explicit_period=off  period=1  equ=P=C  opselect=C  inp2=PCIN>>17  opr=+  inp1=P  carry=CIN  dbl_ovrd=off  block_type=constant
  Constant4 : xbsIndex_r4/Constant  const=6  gui_display_data_type=Fixed-point  arith_type=Unsigned  n_bits=3  bin_pt=0  preci_type=Single  exp_width=8  frac_width=24  explicit_period=off  period=1  equ=P=C  opselect=C  inp2=PCIN>>17  opr=+  inp1=P  carry=CIN  dbl_ovrd=off  block_type=constant
  Constant5 : xbsIndex_r4/Constant  const=2  gui_display_data_type=Fixed-point  arith_type=Unsigned  n_bits=2  bin_pt=0  preci_type=Single  exp_width=8  frac_width=24  explicit_period=off  period=1  equ=P=C  opselect=C  inp2=PCIN>>17  opr=+  inp1=P  carry=CIN  dbl_ovrd=off  block_type=constant
  Convert : xbsIndex_r4/Convert  gui_display_data_type=Boolean  arith_type=Boolean  n_bits=16  bin_pt=14  float_type=Single  exp_bits=8  fraction_bits=24  quantization=Truncate  overflow=Wrap  en=off  latency=0  dbl_ovrd=off  pipeline=off  block_type=convert
  Demod Config : Subsystem
  Logical : xbsIndex_r4/Logical  logical_function=AND  inputs=3  en=off  latency=0  precision=Full  arith_type=Unsigned  n_bits=16  bin_pt=0  align_bp=on  dbl_ovrd=off  block_type=logical
  Logical1 : xbsIndex_r4/Logical  logical_function=OR  inputs=2  en=off  latency=0  precision=Full  arith_type=Unsigned  n_bits=16  bin_pt=0  align_bp=on  dbl_ovrd=off  block_type=logical
  Logical2 : xbsIndex_r4/Logical  logical_function=NAND  inputs=2  en=off  latency=0  precision=Full  arith_type=Unsigned  n_bits=16  bin_pt=0  align_bp=on  dbl_ovrd=off  block_type=logical
  Logical3 : xbsIndex_r4/Logical  logical_function=AND  inputs=2  en=off  latency=0  precision=Full  arith_type=Unsigned  n_bits=16  bin_pt=0  align_bp=on  dbl_ovrd=off  block_type=logical
  Logical4 : xbsIndex_r4/Logical  logical_function=OR  inputs=2  en=off  latency=0  precision=Full  arith_type=Unsigned  n_bits=8  bin_pt=2  align_bp=on  dbl_ovrd=off  block_type=logical
      settings: xl_area=[1 0 0 1 0 0 0]  block_version=VER_STRING_GOES_HERE
  Mux : xbsIndex_r4/Mux  inputs=2  en=off  latency=0  precision=Full  arith_type=Unsigned  n_bits=16  bin_pt=14  quantization=Truncate  overflow=Wrap  dbl_ovrd=off  block_type=mux
  Posedge : Subsystem
  Posedge1 : Subsystem
  Register : xbsIndex_r4/Register  init=0  rst=off  en=on  dbl_ovrd=off  block_type=register
  Relational1 : xbsIndex_r4/Relational  mode=a>b  en=off  latency=0  dbl_ovrd=off  block_type=relational
  Relational2 : xbsIndex_r4/Relational  mode=a=b  en=off  latency=0  dbl_ovrd=off  block_type=relational
  Relational3 : xbsIndex_r4/Relational  mode=a=b  en=off  latency=0  dbl_ovrd=off  block_type=relational
  Relational4 : xbsIndex_r4/Relational  mode=a=b  en=off  latency=0  dbl_ovrd=off  block_type=relational
  Relational5 : xbsIndex_r4/Relational  mode=a>b  en=off  latency=0  dbl_ovrd=off  block_type=relational
  Relational6 : xbsIndex_r4/Relational  mode=a>b  en=off  latency=0  dbl_ovrd=off  block_type=relational
  SC Sym Map 11a : xbsIndex_r4/ROM  depth=MAX_NUM_SC  initVector=sc_data_sym_map_11a_bool  distributed_mem=Distributed memory  rst=off  init_reg=0  en=off  latency=0  gui_display_data_type=Boolean  arith_type=Boolean  n_bits=ceil(log2(MAX_NUM_SC))+1  bin_pt=0  preci_type=Single  exp_width=8  frac_width=24  dbl_ovrd=off  optimize=Area  use_rpm=on  block_type=sprom
  SC Sym Map 11n/ac : xbsIndex_r4/ROM  depth=MAX_NUM_SC  initVector=sc_data_sym_map_11n_bool  distributed_mem=Distributed memory  rst=off  init_reg=0  en=off  latency=0  gui_display_data_type=Boolean  arith_type=Boolean  n_bits=ceil(log2(MAX_NUM_SC))+1  bin_pt=0  preci_type=Single  exp_width=8  frac_width=24  dbl_ovrd=off  optimize=Area  use_rpm=on  block_type=sprom
  Data I : Outport
  Data Q : Outport  Port=2
  data_sc : Outport  Port=3
  Demod cfg : Outport  Port=4
  Reset Demod : Outport  Port=5
  Start Decoder : Outport  Port=6
  wires:
  sc_ind/1 -> SC Sym Map 11a/1, SC Sym Map 11n/ac/1, Demod Config/1(sc_ind), Relational2/2
  SC Sym Map 11a/1 -> Mux/2
  iq_valid/1 -> Convert/1
  Convert/1 -> Logical/3
  Mux/1 -> Logical/2
  SC Sym Map 11n/ac/1 -> Mux/3
  Register/1 -> Logical2/2, Logical3/1
  Logical/1 -> data_sc/1
  Constant3/1 -> Relational3/1
  Constant4/1 -> Relational4/1, Relational1/2
  Sym ind/1 -> Relational3/2, Relational4/2, Relational1/1, Relational5/1, Relational6/1, Demod Config/2(sym_ind)
  Relational3/1 -> Logical1/1
  Relational4/1 -> Logical1/2
  Logical1/1 -> Logical2/1
  Logical2/1 -> Logical/1
  Logical3/1 -> Mux/1
  Relational1/1 -> Logical3/2
  Eq I/1 -> Data I/1
  Eq Q/1 -> Data Q/1
  [RX_PHY_MODE_11N_AC] -> Register/1
  Constant1/1 -> Relational2/1
  Relational2/1 -> Register/2
  Constant2/1 -> Relational6/2
  Relational6/1 -> Posedge1/1(D)
  Constant5/1 -> Relational5/2
  Relational5/1 -> Posedge/1(D)
  Posedge1/1(Q) -> Logical4/2
  Posedge/1(Q) -> Start Decoder/1
  Logical4/1 -> Reset Demod/1
  Reset/1 -> Logical4/1
  Concat/1 -> Demod cfg/1
  Demod Config/2(QBPSK Sym) -> Concat/1
  Demod Config/3(SIGNAL Sym) -> Concat/2

== system wlan_phy_rx_pmd/Detect & Decode/Sym FIFO ==
  note: Complex symbols are buffered here, to adjust the EQ output rate
      | to the demod/decode input rate. The EQ produces one symbol per
      | cycle (in bursts). The demod produces two soft bits per cycle. This
      | FIFO stores up to one OFDM symbol of IQ samples, allowing even
      | high-order (many bits-per-symbol) schemes enough time to output
      | all the bits represented by the complex symbols.
  note: Hacky way to force FIFO to fill up a bit
      | before unloading, trying to workaround
      | hw/sim mismatch of un-reproducable
      | single cycle tvalid.out -> 0 when
      |  FIFO is near empty
  tready : Inport
  I : Inport  Port=2
  Q : Inport  Port=3
  tvalid : Inport  Port=4
  Demod cfg : Inport  Port=5
  AXI FIFO : xbsIndex_r4/AXI FIFO  input_depth_axis=128  actual_fifo_depth_label=130  enable_tdata=on  enable_tdest=off  enable_tstrb=off  enable_tready=on  enable_tid=off  enable_tuser=on  enable_tkeep=off  enable_tlast=off  has_aresetn=off  enable_data_counts_axis=on  fifo_implementation_type_axis=Common Clock Block RAM  trim_axipin_name=on  programmable_full_type_axis=Full  full_threshold_assert_value_axis=0  programmable_empty_type_axis=Empty  empty_threshold_assert_value_axis=1022  block_type=axi_fifo
  Concat : xbsIndex_r4/Concat  num_inputs=2  dbl_ovrd=off  block_type=concat
  Constant3 : xbsIndex_r4/Constant  const=20  gui_display_data_type=Fixed-point  arith_type=Unsigned  n_bits=5  bin_pt=0  preci_type=Single  exp_width=8  frac_width=24  explicit_period=off  period=1  equ=P=C  opselect=C  inp2=PCIN>>17  opr=+  inp1=P  carry=CIN  dbl_ovrd=off  block_type=constant
  Constant4 : xbsIndex_r4/Constant  const=0  gui_display_data_type=Fixed-point  arith_type=Unsigned  n_bits=5  bin_pt=0  preci_type=Single  exp_width=8  frac_width=24  explicit_period=off  period=1  equ=P=C  opselect=C  inp2=PCIN>>17  opr=+  inp1=P  carry=CIN  dbl_ovrd=off  block_type=constant
  Convert : xbsIndex_r4/Convert  gui_display_data_type=Fixed-point  arith_type="Signed  (2's comp)"  n_bits=8  bin_pt=7  float_type=Single  exp_bits=8  fraction_bits=24  quantization=Truncate  overflow=Saturate  en=off  latency=1  dbl_ovrd=off  pipeline=off  block_type=convert
  Convert2 : xbsIndex_r4/Convert  gui_display_data_type=Fixed-point  arith_type="Signed  (2's comp)"  n_bits=8  bin_pt=7  float_type=Single  exp_bits=8  fraction_bits=24  quantization=Truncate  overflow=Saturate  en=off  latency=1  dbl_ovrd=off  pipeline=off  block_type=convert
  Delay : xbsIndex_r4/Delay  rst=off  infoeditControl=Selection of Reset will increase slice count due to use of real FFs and instead of SRLs  en=off  latency=1  dbl_ovrd=off  reg_retiming=off  block_type=delay
  Delay1 : xbsIndex_r4/Delay  rst=off  infoeditControl=Selection of Reset will increase slice count due to use of real FFs and instead of SRLs  en=off  latency=1  dbl_ovrd=off  reg_retiming=off  block_type=delay
  Gateway Out{1..10} (10 alike) : xbsIndex_r4/Gateway Out  inherit_from_input=off  hdl_port=off  timing_constraint=None  locs_specified=off  LOCs={}  UseAsDAC=off  DACChannel='1'  block_type=gatewayout
  Logical1 : xbsIndex_r4/Logical  logical_function=AND  inputs=2  en=off  latency=0  precision=Full  arith_type=Unsigned  n_bits=16  bin_pt=0  align_bp=on  dbl_ovrd=off  block_type=logical
  Logical2 : xbsIndex_r4/Logical  logical_function=AND  inputs=2  en=off  latency=0  precision=Full  arith_type=Unsigned  n_bits=16  bin_pt=0  align_bp=on  dbl_ovrd=off  block_type=logical
  Reinterpret : xbsIndex_r4/Reinterpret  force_arith_type=on  arith_type=Unsigned  force_bin_pt=on  bin_pt=0  block_type=reinterpret
  Reinterpret1 : xbsIndex_r4/Reinterpret  force_arith_type=on  arith_type="Signed  (2's comp)"  force_bin_pt=on  bin_pt=7  block_type=reinterpret
  Reinterpret3 : xbsIndex_r4/Reinterpret  force_arith_type=on  arith_type="Signed  (2's comp)"  force_bin_pt=on  bin_pt=7  block_type=reinterpret
  Reinterpret4 : xbsIndex_r4/Reinterpret  force_arith_type=on  arith_type=Unsigned  force_bin_pt=on  bin_pt=0  block_type=reinterpret
  Relational3 : xbsIndex_r4/Relational  mode=a>=b  en=off  latency=0  dbl_ovrd=off  block_type=relational
  Relational4 : xbsIndex_r4/Relational  mode=a=b  en=off  latency=0  dbl_ovrd=off  block_type=relational
  S-R Latch1 : Subsystem
  Slice : xbsIndex_r4/Slice  nbits=8  boolean_output=off  mode=Lower Bit Location + Width  bit1=0  base1=MSB of Input  bit0=0  base0=LSB of Input  dbl_ovrd=off  block_type=slice
  Slice1 : xbsIndex_r4/Slice  nbits=8  boolean_output=off  mode=Lower Bit Location + Width  bit1=0  base1=MSB of Input  bit0=8  base0=LSB of Input  dbl_ovrd=off  block_type=slice
  Sym FIFO : Scope  NumInputPorts=10
      settings: TimeRange=20000  YMin=0~-1~0~0~-1~0~0~0~0~-5  YMax=1~1~1~1~1~1~1~5~30~5
  " tvalid" : Outport
  " I" : Outport  Port=2
  " Q" : Outport  Port=3
  " Demod cfg" : Outport  Port=4
  wires:
  Concat/1 -> AXI FIFO/3
  Slice1/1 -> Reinterpret1/1
  Slice/1 -> Reinterpret3/1
  Logical1/1 -> AXI FIFO/1, Gateway Out4/1
  Reinterpret1/1 -> " I"/1, Gateway Out5/1
  Reinterpret3/1 -> " Q"/1
  tvalid/1 -> Delay/1, Gateway Out2/1
  I/1 -> Convert/1, Gateway Out3/1
  Q/1 -> Convert2/1
  Convert/1 -> Reinterpret/1
  Reinterpret/1 -> Concat/1
  Convert2/1 -> Reinterpret4/1
  Reinterpret4/1 -> Concat/2
  Delay/1 -> AXI FIFO/2
  Gateway Out2/1 -> Sym FIFO/1  "Src Valid"
  Gateway Out3/1 -> Sym FIFO/2  "Src I"
  Gateway Out4/1 -> Sym FIFO/3  "Sink Ready"
  Gateway Out1/1 -> Sym FIFO/4  "Out valid"
  Gateway Out5/1 -> Sym FIFO/5  "Out I"
  Gateway Out6/1 -> Sym FIFO/6  "tuser out"
  Demod cfg/1 -> Delay1/1, Gateway Out8/1
  AXI FIFO/3 -> " Demod cfg"/1, Gateway Out6/1
  Gateway Out7/1 -> Sym FIFO/7  "tuser in"
  Gateway Out8/1 -> Sym FIFO/8  "OFDM sym ind"
  AXI FIFO/5 -> Relational3/1, Relational4/1, Gateway Out9/1
  Gateway Out9/1 -> Sym FIFO/9  "FIFO Occ"
  AXI FIFO/1 -> Logical2/1, Gateway Out1/1
  AXI FIFO/2 -> Slice1/1, Slice/1
  Gateway Out10/1 -> Sym FIFO/10  "Ready for input"
  Logical2/1 -> " tvalid"/1
  Constant3/1 -> Relational3/2
  Relational3/1 -> S-R Latch1/1(S)
  S-R Latch1/1(Q) -> Logical2/2, Logical1/1
  Constant4/1 -> Relational4/2
  Relational4/1 -> S-R Latch1/2(R)
  tready/1 -> Logical1/2
  Delay1/1 -> AXI FIFO/4, Gateway Out7/1
  AXI FIFO/4 -> Gateway Out10/1
